simulink model "SnsrTEstimnForCmptmt"  (R2022b)
settings: Solver=FixedStepDiscrete  StartTime=0.0  StopTime=10.0  FixedStep=1  RelTol=1e-3
settings Model: Creator=blue.ge  LastModifiedBy=blue.ge
settings DataIOCC: SaveFormat=StructureWithTime
settings OptimizationCC: DefaultParameterBehavior=Inlined  EnhancedBackFolding=on  PassReuseOutputArgsAs=Individual arguments  ZeroExternalMemoryAtStartup=off  ZeroInternalMemoryAtStartup=off  OptimizeBlockOrder=speed  DifferentSizesBufferReuse=on  OptimizationCustomize=off  EfficientTunableParamExpr=on
settings DebuggingCC: ParameterTunabilityLossMsg=error
settings ModelReferenceCC: ModelReferenceNumInstancesAllowed=Single
settings SFSimCC: MATLABDynamicMemAlloc=off
settings RTWCC: Description=AUTOSAR  SystemTargetFile=autosar.tlc  GenCodeOnly=on  TemplateMakefile=ert_default_tmf
settings CodeAppCC: ForceParamTrailComments=on  IgnoreCustomStorageClasses=off  OperatorAnnotations=on  SimulinkDataObjDesc=on  SFDataObjDesc=on  InsertBlockDesc=on
settings STFCustomTargetCC: TargetLangStandard=C99 (ISO)  MultiwordLength=256  ParMdlRefBuildCompliant=on  CompOptLevelCompliant=on  IncludeMdlTerminateFcn=off  CombineOutputUpdateFcns=on  SuppressErrorStatus=on  ERTCustomFileBanners=on  SupportNonFinite=off  SupportComplex=off  SupportContinuousTime=off  SupportNonInlinedSFcns=off  RemoveResetFunc=on  MATLABClassNameForMDSCustomization=Simulink.SoftwareTarget.ERTCustomization  AutosarCompliant=on  UseToolchainInfoCompliant=on  LookupTableObjectStructAxisOrder=2,1,3,4,...  LUTObjectStructOrderEvenSpacing=Size,Table,Breakpoints  SystemTargetFile=autosar.tlc
settings ERTTargetCC: TargetLangStandard=C99 (ISO)  ConcurrentExecutionCompliant=off  IncludeMdlTerminateFcn=off  SuppressErrorStatus=on  SupportNonFinite=off  SupportComplex=off  ModelStepFunctionPrototypeControlCompliant=off  CPPClassGenCompliant=off  AutosarCompliant=on  LookupTableObjectStructAxisOrder=2,1,3,4,...  LUTObjectStructOrderEvenSpacing=Size,Table,Breakpoints  InlinedParameterPlacement=NonHierarchical  ERTCustomFileTemplate=example_file_process.tlc

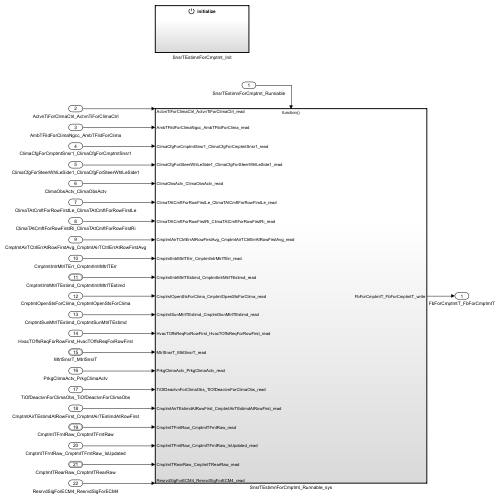
[thumbnail: Simulink's stored preview of the top-level diagram]
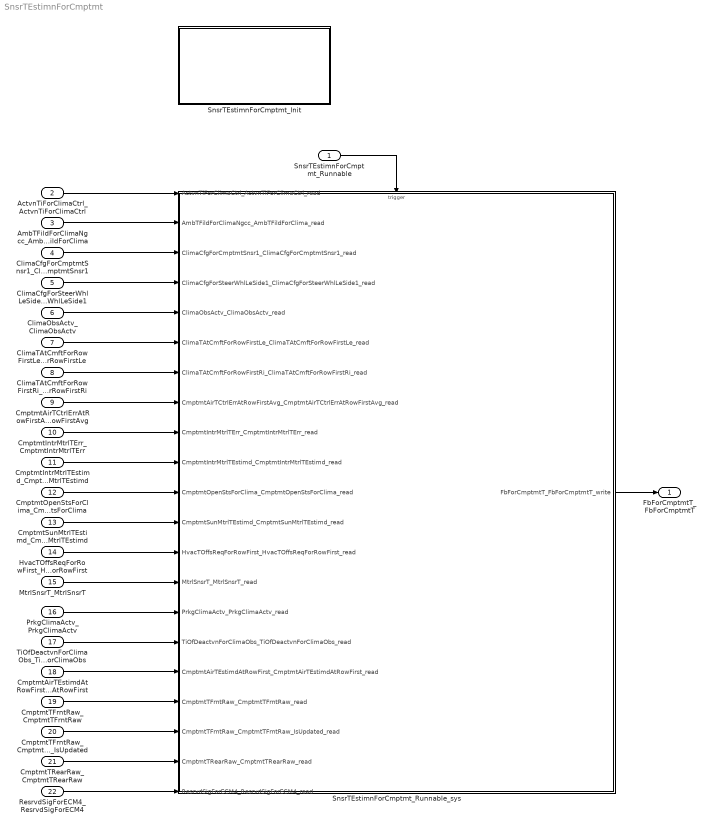
== system SnsrTEstimnForCmptmt ==
  SnsrTEstimnForCmptmt_Runnable : Inport  SampleTime=1  OutputFunctionCall=on
  ActvnTiForClimaCtrl_ActvnTiForClimaCtrl : Inport  Port=2  OutDataTypeStr=Ti33  PortDimensions=1
  AmbTFildForClimaNgcc_AmbTFildForClima : Inport  Port=3  OutDataTypeStr=T30  PortDimensions=1
  ClimaCfgForCmptmtSnsr1_ClimaCfgForCmptmtSnsr1 : Inport  Port=4  OutDataTypeStr=Enum: ClimaCfgForCmptmtSnsr1  PortDimensions=1
  ClimaCfgForSteerWhlLeSide1_ClimaCfgForSteerWhlLeSide1 : Inport  Port=5  OutDataTypeStr=Boolean  PortDimensions=1
  ClimaObsActv_ClimaObsActv : Inport  Port=6  OutDataTypeStr=Boolean  PortDimensions=1
  ClimaTAtCmftForRowFirstLe_ClimaTAtCmftForRowFirstLe : Inport  Port=7  OutDataTypeStr=T30  PortDimensions=1
  ClimaTAtCmftForRowFirstRi_ClimaTAtCmftForRowFirstRi : Inport  Port=8  OutDataTypeStr=T30  PortDimensions=1
  CmptmtAirTCtrlErrAtRowFirstAvg_CmptmtAirTCtrlErrAtRowFirstAvg : Inport  Port=9  OutDataTypeStr=T30  PortDimensions=1
  CmptmtIntrMtrlTErr_CmptmtIntrMtrlTErr : Inport  Port=10  OutDataTypeStr=T30  PortDimensions=1
  CmptmtIntrMtrlTEstimd_CmptmtIntrMtrlTEstimd : Inport  Port=11  OutDataTypeStr=Bus: MtrlSnsrT  PortDimensions=1  BusOutputAsStruct=on
  CmptmtOpenStsForClima_CmptmtOpenStsForClima : Inport  Port=12  OutDataTypeStr=Boolean  PortDimensions=1
  CmptmtSunMtrlTEstimd_CmptmtSunMtrlTEstimd : Inport  Port=13  OutDataTypeStr=T30  PortDimensions=1
  HvacTOffsReqForRowFirst_HvacTOffsReqForRowFirst : Inport  Port=14  OutDataTypeStr=T30  PortDimensions=1
  MtrlSnsrT_MtrlSnsrT : Inport  Port=15  OutDataTypeStr=Bus: MtrlSnsrT  PortDimensions=1  BusOutputAsStruct=on
  PrkgClimaActv_PrkgClimaActv : Inport  Port=16  OutDataTypeStr=Enum: PrkgClimaActv  PortDimensions=1
  TiOfDeactvnForClimaObs_TiOfDeactvnForClimaObs : Inport  Port=17  OutDataTypeStr=Ti33  PortDimensions=1
  CmptmtAirTEstimdAtRowFirst_CmptmtAirTEstimdAtRowFirst : Inport  Port=18  OutDataTypeStr=T30  PortDimensions=1
  CmptmtTFrntRaw_CmptmtTFrntRaw : Inport  Port=19  OutDataTypeStr=Bus: CmptmtTFrnt  PortDimensions=1  BusOutputAsStruct=on
  CmptmtTFrntRaw_CmptmtTFrntRaw_IsUpdated : Inport  Port=20  OutDataTypeStr=boolean  PortDimensions=1
  CmptmtTRearRaw_CmptmtTRearRaw : Inport  Port=21  OutDataTypeStr=Bus: CmptmtTFrnt  PortDimensions=1  BusOutputAsStruct=on
  ResrvdSigForECM4_ResrvdSigForECM4 : Inport  Port=22  OutDataTypeStr=BattSnsrChksTbl  PortDimensions=1
  SnsrTEstimnForCmptmt_Init : Subsystem
  SnsrTEstimnForCmptmt_Runnable_sys : Subsystem
  FbForCmptmtT_FbForCmptmtT : Outport  OutDataTypeStr=T30  PortDimensions=1
  wires:
  ActvnTiForClimaCtrl_ActvnTiForClimaCtrl/1 -> SnsrTEstimnForCmptmt_Runnable_sys/1(ActvnTiForClimaCtrl_ActvnTiForClimaCtrl_read)
  AmbTFildForClimaNgcc_AmbTFildForClima/1 -> SnsrTEstimnForCmptmt_Runnable_sys/2(AmbTFildForClimaNgcc_AmbTFildForClima_read)
  ClimaCfgForCmptmtSnsr1_ClimaCfgForCmptmtSnsr1/1 -> SnsrTEstimnForCmptmt_Runnable_sys/3(ClimaCfgForCmptmtSnsr1_ClimaCfgForCmptmtSnsr1_read)
  ClimaCfgForSteerWhlLeSide1_ClimaCfgForSteerWhlLeSide1/1 -> SnsrTEstimnForCmptmt_Runnable_sys/4(ClimaCfgForSteerWhlLeSide1_ClimaCfgForSteerWhlLeSide1_read)
  ClimaObsActv_ClimaObsActv/1 -> SnsrTEstimnForCmptmt_Runnable_sys/5(ClimaObsActv_ClimaObsActv_read)
  ClimaTAtCmftForRowFirstLe_ClimaTAtCmftForRowFirstLe/1 -> SnsrTEstimnForCmptmt_Runnable_sys/6(ClimaTAtCmftForRowFirstLe_ClimaTAtCmftForRowFirstLe_read)
  ClimaTAtCmftForRowFirstRi_ClimaTAtCmftForRowFirstRi/1 -> SnsrTEstimnForCmptmt_Runnable_sys/7(ClimaTAtCmftForRowFirstRi_ClimaTAtCmftForRowFirstRi_read)
  CmptmtAirTCtrlErrAtRowFirstAvg_CmptmtAirTCtrlErrAtRowFirstAvg/1 -> SnsrTEstimnForCmptmt_Runnable_sys/8(CmptmtAirTCtrlErrAtRowFirstAvg_CmptmtAirTCtrlErrAtRowFirstAvg_read)
  CmptmtIntrMtrlTErr_CmptmtIntrMtrlTErr/1 -> SnsrTEstimnForCmptmt_Runnable_sys/9(CmptmtIntrMtrlTErr_CmptmtIntrMtrlTErr_read)
  CmptmtIntrMtrlTEstimd_CmptmtIntrMtrlTEstimd/1 -> SnsrTEstimnForCmptmt_Runnable_sys/10(CmptmtIntrMtrlTEstimd_CmptmtIntrMtrlTEstimd_read)
  CmptmtOpenStsForClima_CmptmtOpenStsForClima/1 -> SnsrTEstimnForCmptmt_Runnable_sys/11(CmptmtOpenStsForClima_CmptmtOpenStsForClima_read)
  CmptmtSunMtrlTEstimd_CmptmtSunMtrlTEstimd/1 -> SnsrTEstimnForCmptmt_Runnable_sys/12(CmptmtSunMtrlTEstimd_CmptmtSunMtrlTEstimd_read)
  HvacTOffsReqForRowFirst_HvacTOffsReqForRowFirst/1 -> SnsrTEstimnForCmptmt_Runnable_sys/13(HvacTOffsReqForRowFirst_HvacTOffsReqForRowFirst_read)
  MtrlSnsrT_MtrlSnsrT/1 -> SnsrTEstimnForCmptmt_Runnable_sys/14(MtrlSnsrT_MtrlSnsrT_read)
  PrkgClimaActv_PrkgClimaActv/1 -> SnsrTEstimnForCmptmt_Runnable_sys/15(PrkgClimaActv_PrkgClimaActv_read)
  TiOfDeactvnForClimaObs_TiOfDeactvnForClimaObs/1 -> SnsrTEstimnForCmptmt_Runnable_sys/16(TiOfDeactvnForClimaObs_TiOfDeactvnForClimaObs_read)
  CmptmtAirTEstimdAtRowFirst_CmptmtAirTEstimdAtRowFirst/1 -> SnsrTEstimnForCmptmt_Runnable_sys/17(CmptmtAirTEstimdAtRowFirst_CmptmtAirTEstimdAtRowFirst_read)
  CmptmtTFrntRaw_CmptmtTFrntRaw/1 -> SnsrTEstimnForCmptmt_Runnable_sys/18(CmptmtTFrntRaw_CmptmtTFrntRaw_read)
  CmptmtTFrntRaw_CmptmtTFrntRaw_IsUpdated/1 -> SnsrTEstimnForCmptmt_Runnable_sys/19(CmptmtTFrntRaw_CmptmtTFrntRaw_IsUpdated_read)
  CmptmtTRearRaw_CmptmtTRearRaw/1 -> SnsrTEstimnForCmptmt_Runnable_sys/20(CmptmtTRearRaw_CmptmtTRearRaw_read)
  ResrvdSigForECM4_ResrvdSigForECM4/1 -> SnsrTEstimnForCmptmt_Runnable_sys/21(ResrvdSigForECM4_ResrvdSigForECM4_read)
  SnsrTEstimnForCmptmt_Runnable_sys/1(FbForCmptmtT_FbForCmptmtT_write) -> FbForCmptmtT_FbForCmptmtT/1
  SnsrTEstimnForCmptmt_Runnable/1 -> SnsrTEstimnForCmptmt_Runnable_sys/Trigger

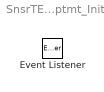
== system SnsrTEstimnForCmptmt/SnsrTEstimnForCmptmt_Init ==
  Event Listener : EventListener  EventName=reset

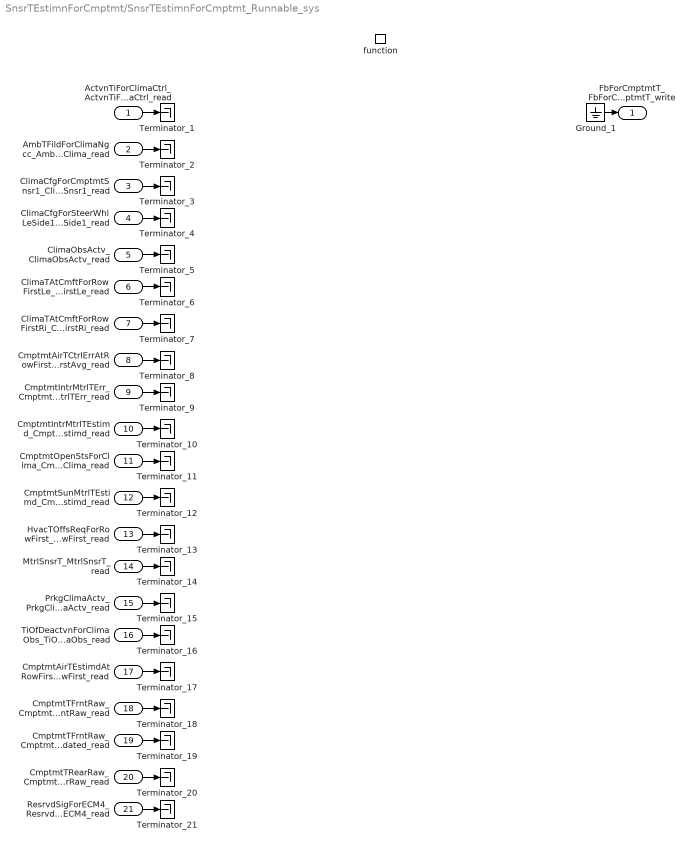
== system SnsrTEstimnForCmptmt/SnsrTEstimnForCmptmt_Runnable_sys ==
  ActvnTiForClimaCtrl_ActvnTiForClimaCtrl_read : Inport
  AmbTFildForClimaNgcc_AmbTFildForClima_read : Inport  Port=2
  ClimaCfgForCmptmtSnsr1_ClimaCfgForCmptmtSnsr1_read : Inport  Port=3
  ClimaCfgForSteerWhlLeSide1_ClimaCfgForSteerWhlLeSide1_read : Inport  Port=4
  ClimaObsActv_ClimaObsActv_read : Inport  Port=5
  ClimaTAtCmftForRowFirstLe_ClimaTAtCmftForRowFirstLe_read : Inport  Port=6
  ClimaTAtCmftForRowFirstRi_ClimaTAtCmftForRowFirstRi_read : Inport  Port=7
  CmptmtAirTCtrlErrAtRowFirstAvg_CmptmtAirTCtrlErrAtRowFirstAvg_read : Inport  Port=8
  CmptmtIntrMtrlTErr_CmptmtIntrMtrlTErr_read : Inport  Port=9
  CmptmtIntrMtrlTEstimd_CmptmtIntrMtrlTEstimd_read : Inport  Port=10
  CmptmtOpenStsForClima_CmptmtOpenStsForClima_read : Inport  Port=11
  CmptmtSunMtrlTEstimd_CmptmtSunMtrlTEstimd_read : Inport  Port=12
  HvacTOffsReqForRowFirst_HvacTOffsReqForRowFirst_read : Inport  Port=13
  MtrlSnsrT_MtrlSnsrT_read : Inport  Port=14
  PrkgClimaActv_PrkgClimaActv_read : Inport  Port=15
  TiOfDeactvnForClimaObs_TiOfDeactvnForClimaObs_read : Inport  Port=16
  CmptmtAirTEstimdAtRowFirst_CmptmtAirTEstimdAtRowFirst_read : Inport  Port=17
  CmptmtTFrntRaw_CmptmtTFrntRaw_read : Inport  Port=18
  CmptmtTFrntRaw_CmptmtTFrntRaw_IsUpdated_read : Inport  Port=19
  CmptmtTRearRaw_CmptmtTRearRaw_read : Inport  Port=20
  ResrvdSigForECM4_ResrvdSigForECM4_read : Inport  Port=21
  function : TriggerPort  TriggerType=function-call  VariantControl=(inherit)  SampleTimeType=periodic  SampleTime=-1
  Ground_1 : Ground
  Terminator_{1..21} (21 alike) : Terminator
  FbForCmptmtT_FbForCmptmtT_write : Outport
      settings: EnsureOutportIsVirtual=on
  wires:
  ActvnTiForClimaCtrl_ActvnTiForClimaCtrl_read/1 -> Terminator_1/1
  AmbTFildForClimaNgcc_AmbTFildForClima_read/1 -> Terminator_2/1
  ClimaCfgForCmptmtSnsr1_ClimaCfgForCmptmtSnsr1_read/1 -> Terminator_3/1
  ClimaCfgForSteerWhlLeSide1_ClimaCfgForSteerWhlLeSide1_read/1 -> Terminator_4/1
  ClimaObsActv_ClimaObsActv_read/1 -> Terminator_5/1
  ClimaTAtCmftForRowFirstLe_ClimaTAtCmftForRowFirstLe_read/1 -> Terminator_6/1
  ClimaTAtCmftForRowFirstRi_ClimaTAtCmftForRowFirstRi_read/1 -> Terminator_7/1
  CmptmtAirTCtrlErrAtRowFirstAvg_CmptmtAirTCtrlErrAtRowFirstAvg_read/1 -> Terminator_8/1
  CmptmtIntrMtrlTErr_CmptmtIntrMtrlTErr_read/1 -> Terminator_9/1
  CmptmtIntrMtrlTEstimd_CmptmtIntrMtrlTEstimd_read/1 -> Terminator_10/1
  CmptmtOpenStsForClima_CmptmtOpenStsForClima_read/1 -> Terminator_11/1
  CmptmtSunMtrlTEstimd_CmptmtSunMtrlTEstimd_read/1 -> Terminator_12/1
  HvacTOffsReqForRowFirst_HvacTOffsReqForRowFirst_read/1 -> Terminator_13/1
  MtrlSnsrT_MtrlSnsrT_read/1 -> Terminator_14/1
  PrkgClimaActv_PrkgClimaActv_read/1 -> Terminator_15/1
  TiOfDeactvnForClimaObs_TiOfDeactvnForClimaObs_read/1 -> Terminator_16/1
  CmptmtAirTEstimdAtRowFirst_CmptmtAirTEstimdAtRowFirst_read/1 -> Terminator_17/1
  CmptmtTFrntRaw_CmptmtTFrntRaw_read/1 -> Terminator_18/1
  CmptmtTFrntRaw_CmptmtTFrntRaw_IsUpdated_read/1 -> Terminator_19/1
  CmptmtTRearRaw_CmptmtTRearRaw_read/1 -> Terminator_20/1
  ResrvdSigForECM4_ResrvdSigForECM4_read/1 -> Terminator_21/1
  Ground_1/1 -> FbForCmptmtT_FbForCmptmtT_write/1
